simulink model "light_utility_aircraft_model"  (R2025b)
settings: Solver=ode23t  StartTime=0.0  StopTime=7800*4000  MaxStep=1000  RelTol=1e-1
values from neighbouring files (symbols this model uses):
  batt_temp = 273+25    (= 298)    [model_parameters.m]
  batt_volt = 647    [model_parameters.m]
  Cch = 3    [model_parameters.m]
  Cdch = 1    [model_parameters.m]
  Crate = 360    [model_parameters.m]
  max_SOC = 100    [model_parameters.m]
  min_SOC = 43    [model_parameters.m]
undefined symbols (not defined in the file or in its folder): battery_builder_rtmsupport, DiscIntNeverNeededParam, gcbh
libraries: nesl_utility x254, cylindricalPack_58kwhr_30Q_fade_lib x120, batt_lib x48, fl_lib x6, dspswit3 x2, simulink x1
(part 1 of 25)
settings Model: Creator=OADEBAJO  LastModifiedBy=oadebajo
settings DebuggingCC: AlgebraicLoopMsg=none

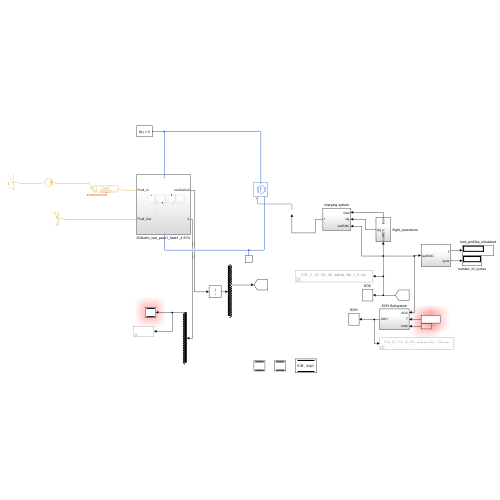
[thumbnail: Simulink's stored preview of the top-level diagram]
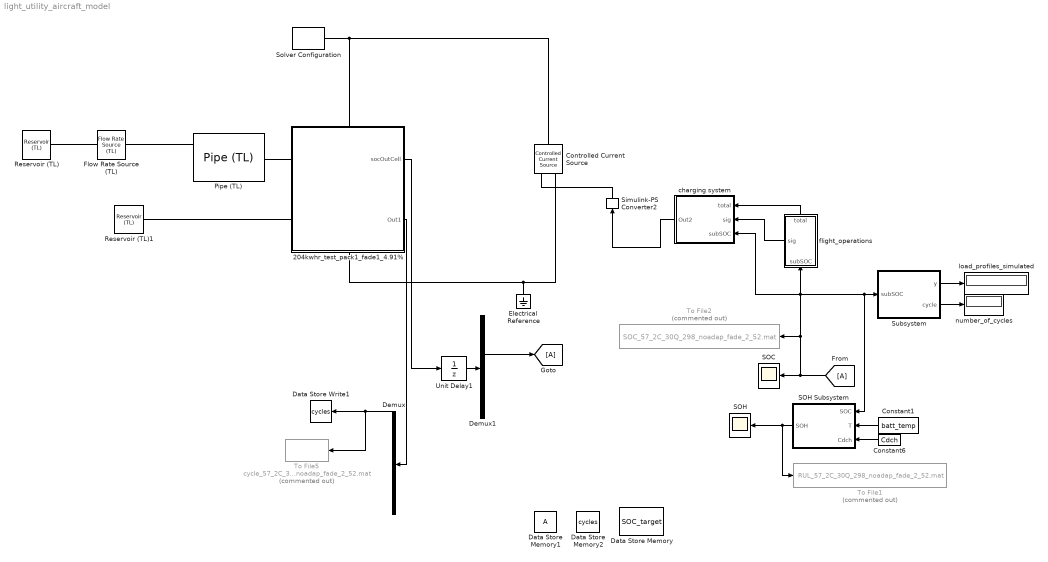
== system light_utility_aircraft_model ==
  204kwhr_test_pack1_fade1_4.91% : Subsystem
      settings: PortSchema="{\"entries\":[{\"content\":{\"sides\":[{\"content\":{\"connectorIds\":[\"LConn1\",\"LConn2\"]},\"type\":\"ConnectorPlacement.EquallySpacedRectSide\",\"uuid\":\"214b9fcb-0c97-4a4b-90ce-45ac7c4dd314\"},{\"content\":{\"connectorIds\":[\"Out1\",\"Out2\"],\"side\":\"RIGHT\"},\"type\":\"ConnectorPlacement.EquallySpacedRectSide\",\"uuid\":\"907ae7f8-29a0-40ea-b941-2e50330af598\"},{\"content\":{\"connectorIds\":[\"LConn3\"],\"side\":\"TOP\"},\"type\":\"ConnectorPlacement.EquallySpacedRectSide\",\"uuid\":\"96475e4e-0276-4503-89b2-fbbc8f8b3a57\"},{\"content\":{\"connectorIds\":[\"RConn1\"],\"side\":\"BOTTOM\"},\"type\":\"ConnectorPlacement.EquallySpacedRectSide\",\"uuid\":\"b4d79bed-ba89-429f-9016-8e0b58ea1225\"}]},\"type\":\"ConnectorPlacement.EquallySpacedConnector\",\"uuid\":\"7f0a58fe-ee0d-4bec-96b9-f1a67c994a61\"}],\"packageUris\":[\"http://schema.mathworks.com/mf0/Connector/R2020b\"],\"version\":\"1.0\"}"
  Constant1 : Constant  Value=batt_temp
  Constant6 : Constant  Value=Cdch
  Controlled Current Source : fl_lib/Electrical/Electrical Sources/Controlled Current Source
  Data Store Memory : DataStoreMemory  DataStoreName=SOC_target  WriteAfterWriteMsg=warning  ShowAdditionalParam=off
  Data Store Memory1 : DataStoreMemory  ReadBeforeWriteMsg=warning  WriteAfterWriteMsg=warning  WriteAfterReadMsg=warning  InitialValue=|  ShowAdditionalParam=off  OutDataTypeStr=double
      | [2000
      | 38016.66667
      | 37985.83333
      | 38114
      | 38470
      | 38050
      | 36925
      | 36686.66667
      | 36356.66667
      | 36665
      | 36675.83333
      | 36373.33333
      | 36550
      | 36696.66667
      | 36866.66667
      | 37022.5
      | 37191.33333
      | 37345
      | 37218
      | 37385.83333
      | 37170
      | 36890
      | 36601.33333
      | 36200.83333
      | 35166
      | 32305
      | 32462.66667
      | 31617.5
      | 31165.83333
      | 30523.33333
      | 30460
      | 30261.66667
      | 29913.33333
      | 30440
      | 31586.66667
      | 31543.33333
      | 31075
      | 30460
      | 30705
      | 31030
      | 31563.33333
      | 32118
      | 31650
      | 30480
      | 30505.83333
      | 31034
      | 31050
      | 31050
      | 31050
      | 31050
      | 30870
      | 30825
      | 30851.66667
      | 30460
      | 30300
      | 29880
      | 30050
      | 30460
      | 30460
      | 30508.33333
      | 30804
      | 30490
      | 30820
      | 30460
      | 30460
      | 30460
      | 30164
      | 29880
      | 30173.33333
      | 30460
      | 30460
      | 30293.33333
      | 30230
      | 30646.66667
      | 31427.5
      | 32174
      | 31580
      | 30460
      | 30512.5
      | 31060.66667
      | 31136.66667
      | 31200
      | 31283.33333
      | 31357.33333
      | 31430
      | 31504.66667
      | 31577.5
      | 31916
      | 32126.66667
      | 31566.66667
      | 31327.5
      | 31115.83333
      | 30533.33333
      | 30460
      | 30460
      | 30816.66667
      | 31050
      | 31050
      | 31050
      | 31000
      | 30270
      | 31118.33333
      | 31560
      | 31132.5
      | 31809.33333
      | 32210
      | 32210
      | 31656.66667
      | 31357.5
      | 30986.66667
      | 30753.33333
      | 31897.5
      | 31917.5
      | 30770
      | 31050
      | 30644
      | 29657.5
      | 30580
      | 30823.33333
      | 31196.66667
      | 29307.5
      | 29892.5
      | 26681.66667
      | 27240
      | 27540
      | 27362.66667
      | 26960
      | 26960
      | 26960
      | 26960
      | 26960
      | 26960
      | 27465
      | 27540
      | 27036.66667
      | 26960
      | 26960
      | 27295
      | 27540
      | 27540
      | 27396.66667
      | 26960
      | 27047.5
      | 27135
      | 26960
      | 27411.66667
      | 27540
      | 27030
      | 26570.66667
      | 28661.66667
      | 33762.5
      | 39203.33333
      | 60446.66667
      | 82117.5
      | 110829.1667
      | 110388
      | 116205
      | 118564
      | 118603.3333
      | 119512
      | 111716.6667
      | 83120
      | 66835
      | 34828.33333
      | 36093.33333
      | 33270
      | 31767.5
      | 31630
      | 31630
      | 31213.33333
      | 32210
      | 30717.5
      | 30460
      | 30460
      | 30460
      | 30460
      | 30816.66667
      | 30460
      | 30586
      | 31050
      | 30910
      | 30490
      | 30938.33333
      | 30460
      | 30528.33333
      | 30651.66667
      | 30560
      | 31025
      | 30479.33333
      | 30460
      | 30460
      | 30460
      | 30147.5
      | 30501.66667
      | 31023.33333
      | 30596
      | 30460
      | 30561.33333
      | 30755
      | 29981.33333
      | 29880
      | 29880
      | 29903.33333
      | 30418.33333
      | 30817.5
      | 30991.66667
      | 30460
      | 30460
      | 30460
      | 30460
      | 30950
      | 31050
      | 31050
      | 31050
      | 31050
      | 31050
      | 30600
      | 30460
      | 30818
      | 30460
      | 30816.66667
      | 31089.16667
      | 30940
      | 31676.66667
      | 31516.66667
      | 30427.5
      | 29880
      | 30251.66667
      | 30460
      | 30442
      | 29905.83333
      | 30350
      | 31010
      | 30538.33333
      | 30460
      | 31313.33333
      | 32093.33333
      | 33714
      | 34307.5
      | 33970
      | 33695
      | 33818.66667
      | 34481.66667
      | 34820
      | 59860
      | 76430
      | 151665
      | 154560.8333
      | 232958.6667
      | 231350
      | 232070
      | 231858.3333
      | 220890
      | 225815
      | 229102.5
      | 230615
      | 232486.6667
      | 233333.3333
      | 233500
      | 232154.1667
      | 230468
      | 228930
      | 229006.6667
      | 229836.6667
      | 230382.5
      | 223366.6667
      | 216663.3333
      | 204277.5
      | 189920.8333
      | 189770
      | 191450
      | 189118.6667
      | 189555.8333
      | 188840
      | 184448.3333
      | 183048.3333
      | 184440
      | 184956.6667
      | 184896.6667
      | 183412
      | 182658.3333
      | 179545.3333
      | 175645
      | 175086.6667
      | 170024.1667
      | 166452.5
      | 163130
      | 165680
      | 161725
      | 160212.5
      | 160422
      | 169190
      | 167867.3333
      | 168483.3333
      | 168900
      | 168465
      | 167872.6667
      | 166057.5
      | 166100
      | 168700
      | 167844
      | 168100
      | 167290
      | 167490
      | 165093.3333
      | 168485
      | 168115
      | 168623.3333
      | 169350
      | 171222
      | 171535
      | 171806.6667
      | 173960
      | 176183.3333
      | 176946.6667
      | 176810
      | 170711.6667
      | 172366.6667
      | 175735
      | 174870
      | 173703.3333
      | 173380
      | 174165.8333
      | 175250
      | 175250
      | 174833.3333
      | 174660
      | 174910
      | 174290
      | 173840
      | 173490
      | 173210.8333
      | 173260
      | 175740
      | 173692.6667
      | 172180.8333
      | 172418
      | 172330
      | 174247.3333
      | 174010
      | 174496.6667
      | 174240
      | 173790
      | 174280.8333
      | 173590
      | 174080
      | 174016.6667
      | 173242.5
      | 172537.5
      | 172866
      | 174051.6667
      | 174064
      | 173778.3333
      | 173918.6667
      | 174060
      | 173563.3333
      | 173038.3333
      | 172910
      | 173450
      | 173620
      | 173922.5
      | 172150
      | 174340
      | 174605
      | 174697.5
      | 174660
      | 174220
      | 174380
      | 174509.1667
      | 175035
      | 177580
      | 177213.3333
      | 173662
      | 174173.3333
      | 173629.3333
      | 173200
      | 173507.3333
      | 172515
      | 171608
      | 172198.3333
      | 173000.6667
      | 173232.5
      | 173450
      | 173680
      | 173885
      | 173599.1667
      | 173805
      | 175200
      | 173950
      | 173506.6667
      | 173697.5
      | 175117.3333
      | 175143.3333
      | 174102
      | 171750
      | 173811.3333
      | 174660
      | 174660.6667
      | 175020.8333
      | 173026
      | 173011.6667
      | 172330
      | 171440
      | 172716.6667
      | 172840
      | 172850
      | 173114.1667
      | 173911.6667
      | 174890
      | 174546.6667
      | 174070
      | 174195
      | 174638
      | 173948.3333
      | 174486
      | 174006.6667
      | 174064
      | 173510
      | 174560
      | 175118.3333
      | 175562
      | 174893.3333
      | 173742.6667
      | 173500
      | 174509.1667
      | 174076.6667
      | 174200
      | 174817.5
      | 174089.1667
      | 174580
      | 175066.6667
      | 174486.6667
      | 174987.5
      | 173500
      | 173693.3333
      | 172910
      | 172910
      | 173134
      | 174060
      | 172480.6667
      | 173487.5
      | 173838
      | 173936.6667
      | 172592.6667
      | 173405
      | 171750
      | 173043.3333
      | 173145
      | 172905.8333
      | 173580.8333
      | 174188
      | 173070
      | 172160
      | 173272.5
      | 174660
      | 173383.3333
      | 172790
      | 173135.8333
      | 172000.6667
      | 174390
      | 174966
      | 173070
      | 173750
      | 173060
      | 173200
      | 172330
      | 174398.3333
      | 175416.6667
      | 176185
      | 176485
      | 174518.3333
      | 174744
      | 172843.3333
      | 173766.6667
      | 173152.5
      | 175450
      | 174283.3333
      | 172910
      | 172915.8333
      | 172345.3333
      | 171750
      | 170495.3333
      | 169069.1667
      | 170326
      | 168965
      | 168830
      | 169212.5
      | 168517.5
      | 168590
      | 169265
      | 169484.6667
      | 169410
      | 169562
      | 168943.3333
      | 168823.3333
      | 168830
      | 168830
      | 168873.3333
      | 169480
      | 169907.5
      | 169426.6667
      | 168680
      | 168102
      | 169845.8333
      | 170252.5
      | 169485
      | 169980
      | 170772.5
      | 169399.1667
      | 168116.6667
      | 167990
      | 168240
      | 171195
      | 171728
      | 171350
      | 170880
      | 169835
      | 169605.8333
      | 167503.3333
      | 168120
      | 167605.8333
      | 168158
      | 169170
      | 168593.3333
      | 170163.3333
      | 173390
      | 173495
      | 173433.3333
      | 172575
      | 172915
      | 173290
      | 173295
      | 173544.6667
      | 172797.5
      | 172496
      | 171420
      | 172030
      | 171930
      | 171915.8333
      | 171133.3333
      | 169760
      | 171856.6667
      | 172175.3333
      | 174600
      | 172131.3333
      | 172020.8333
      | 172120
      | 171860
      | 173156.6667
      | 173735
      | 172939.1667
      | 173216
      | 172055
      | 172908
      | 173535
      | 173430
      | 173166.6667
      | 173220
      | 174190
      | 174660
      | 173180
      | 172650
      | 173004.1667
      | 172119.3333
      | 172910
      | 171673.3333
      | 171375
      | 171712.5
      | 172090
      | 171800
      | 172155
      | 172150
      | 171081.3333
      | 171120
      | 171700
      | 172665
      | 171172
      | 171866.6667
      | 172946.6667
      | 172520
      | 172784.1667
      | 171068.3333
      | 173300
      | 173575
      | 173638
      | 173160
      | 173581.6667
      | 174050.8333
      | 173915
      | 174215
      | 173573.3333
      | 174550
      | 173344.1667
      | 172577.3333
      | 172915
      | 171750
      | 172176.6667
      | 172642
      | 172153.3333
      | 173625
      | 172677.5
      | 172861.6667
      | 171396.6667
      | 171830
      | 172711.3333
      | 173547.5
      | 173300
      | 171933.3333
      | 172330
      | 172330
      | 172296.6667
      | 172510
      | 172276
      | 170840.8333
      | 172425.3333
      | 172650
      | 172637.3333
      | 173182.5
      | 172992.5
      | 173086.6667
      | 172876.6667
      | 173650
      | 173451.6667
      | 174288.3333
      | 174160
      | 174604.6667
      | 174315.8333
      | 171380
      | 172448.3333
      | 173700
      | 172890
      | 171913.3333
      | 172266.6667
      | 172204
      | 172184.1667
      | 172258
      | 172910
      | 173494.6667
      | 171160
      | 171597.5
      | 171380
      | 171338.3333
      | 170945
      | 171848.3333
      | 172528.6667
      | 171610
      | 170841.3333
      | 170914.1667
      | 172100
      | 172710
      | 170332.5
      | 171492.5
      | 172476.6667
      | 172910
      | 173252
      | 172918.3333
      | 172154
      | 172650
      | 171816.6667
      | 172024.1667
      | 171832.5
      | 171346.6667
      | 172776.6667
      | 172442.5
      | 172464.1667
      | 173161.3333
      | 173730
      | 173621.3333
      | 172357.5
      | 171420
      | 172786.6667
      | 172821.6667
      | 171702.5
      | 172596.6667
      | 170980
      | 172530
      | 172431.6667
      | 172250
      | 172330
      | 171826.6667
      | 172000
      | 153380
      | 146213.3333
      | 144631.6667
      | 141415
      | 141926.6667
      | 139505.3333
      | 135980
      | 129564.6667
      | 125999.1667
      | 123940
      | 123438.3333
      | 122295.3333
      | 116815
      | 117846.6667
      | 115203.3333
      | 115708
      | 116565.8333
      | 115455.3333
      | 113510
      | 112726.6667
      | 111845.8333
      | 108307.5
      | 96923.33333
      | 86050
      | 75792
      | 73804.16667
      | 74118.66667
      | 73000
      | 72070
      | 70140.83333
      | 67820
      | 60416.66667
      | 59270
      | 60102.5
      | 59760
      | 59180
      | 58600
      | 61015.83333
      | 64317.5
      | 69340
      | 71306.66667
      | 64695.83333
      | 60487.5
      | 40273.33333
      | 34888.33333
      | 31640
      | 27380
      | 25226
      | 23600
      | 24616
      | 25837.5
      | 26462
      | 26331.66667
      | 26380
      | 26905
      | 26543.33333
      | 26703.33333
      | 26016
      | 27002.5
      | 27540
      | 26730
      | 26573.33333
      | 26652.5
      | 26980
      | 27540
      | 27115
      | 27840
      | 28698.33333
      | 32944
      | 31090
      | 32941.33333
      | 33380
      | 33380
      | 31378.33333
      | 32210
      | 31247.5
      | 28076.66667
      | 28710
      | 28710
      | 27811.66667
      | 28130
      | 28130
      | 27800
      | 28675
      | 28565
      | 28444
      | 28330
      | 28208
      | 28095
      | 27962
      | 27850
      | 27738
      | 27615
      | 27870
      | 28130
      | 28130
      | 28130
      | 27730
      | 27540
      | 27540
      | 27540
      | 27540
      | 27755
      | 28070
      | 27540
      | 27540
      | 27540
      | 27540
      | 27540
      | 27540
      | 16000
      | 2000]
  Data Store Memory2 : DataStoreMemory  DataStoreName=cycles  WriteAfterWriteMsg=warning  ShowAdditionalParam=off
  Data Store Write1 : DataStoreWrite  DataStoreName=cycles  IndexOptions=Index vector (dialog)  Indices=1  OutputSizes=1
  Demux : Demux  Outputs=30
  Demux1 : Demux  Outputs=30
  Electrical Reference : fl_lib/Electrical/Electrical Elements/Electrical Reference
  Flow Rate Source (TL) : fl_lib/Thermal Liquid/Sources/Flow Rate Source (TL)  source_type=foundation.enum.constant_controlled.constant  flow_type=foundation.enum.mass_volumetric_flow.mass  mass_flow=1.75 kg/s  volumetric_flow=0 m^3/s  power_spec=foundation.enum.power_spec.isentropic  area=0.01 m^2
      settings: InternalSimscapePortConfiguration="{\"Bottom\":[],\"Left\":[{\"id\":\"A\",\"label\":\"A\",\"type\":\"foundation.thermal_liquid.thermal_liquid\"}],\"Right\":[{\"id\":\"B\",\"label\":\"B\",\"type\":\"foundation.thermal_liquid.thermal_liquid\"}],\"Top\":[]}"
  From : From
  Goto : Goto
  Pipe (TL) : fl_lib/Thermal Liquid/Elements/Pipe (TL)  length=5 m  area=0.01 m^2  Dh=0.1128 m  length_add=1 m  roughness=15e-6 m  Re_lam=2000  Re_tur=4000  shape_factor=64  Nu_lam=3.66  dynamic_compressibility=true  inertia=false  p0=0.101325 MPa  T0=293.15 K  mdot0=0 kg/s  p_nominal=0.101325 MPa  T_nominal=293.15 K
  Reservoir (TL) : fl_lib/Thermal Liquid/Elements/Reservoir (TL)  pressure_spec=foundation.enum.pressure_spec.atmospheric  reservoir_pressure=0.101325 MPa  reservoir_temperature=298 K  area=0.01 m^2
  Reservoir (TL)1 : fl_lib/Thermal Liquid/Elements/Reservoir (TL)  pressure_spec=foundation.enum.pressure_spec.atmospheric  reservoir_pressure=0.101325 MPa  reservoir_temperature=298 K  area=0.01 m^2
  SOC : Scope
      settings: ActiveDisplayYMinimum=-0.12565653108728628  ActiveDisplayYMaximum=1.1309087797855768  DataLoggingVariableName=ScopeData4
  SOH : Scope
      settings: ActiveDisplayYMinimum=63.077667459608676  ActiveDisplayYMaximum=104.10248139337682  DataLoggingVariableName=ScopeData3
  SOH Subsystem : Subsystem
  Simulink-PS Converter2 : nesl_utility/Simulink-PS Converter  PhysicalDomain=network_engine_domain  SubClassName=ps_input  LeftPortType=input  RightPortType=output  PseudoPeriodic=off  Frequencies=[]  NoiseDistribution=none  NoiseParameters=[]  Unit=1  AffineConversion=off  FilteringAndDerivatives=provide  SimscapeFilterOrder=1  InputFilterTimeConstant=0.001  UdotUserProvided=0
  Solver Configuration : nesl_utility/Solver Configuration  PhysicalDomain=network_engine_domain  SubClassName=solver  LeftPortType=input  RightPortType=generic  Tones=[]  Harmonics=[]  SimulateNoise=off  Profile=off  UseLocalSampling=off  EnableSwitchedLinearOptims=on  FrequencyDomain=off  UseCCode=off  RelTol=0.001  AbsTol=0.001  MinStep=1e-09  ToWorkspace=off  RevertToSquareIcSolve=off  NumHomotopyIterations=100  PhasorMode=off  PhasorModeNumHarmonics=1  ConsistencySolver=NEWTON_XTOL_AFTER_FTOL  IndexReductionMethod=DerivativeReplacement  DoDC=off  ResidualTolerance=1e-09  ConsistencyTolSource=GLOBAL  ConsistencyAbsTol=0.1  ConsistencyRelTol=0.1  ConsistencyTolFactor=0.1  UseLocalSolver=on  LocalSolverChoice=NE_BACKWARD_EULER_ADVANCER  ResolveIndetEquations=on  FunctionEvalNumThread=1  LocalSolverSampleTime=20  DoFixedCost=off  MaxNonlinIter=3  MaxModeIter=2  ComputeImpulses=off  ImpulseIterations=2  LinearAlgebra=auto  LinearAlgebraNumThread=0  EquationFormulation=NE_TIME_EF  DelaysMemoryBudget=1024  AutomaticFiltering=off  FilteringTimeConstant=0.001  PartitionStorageMethod=AS_NEEDED  PartitionMemoryBudget=1024  PartitionMethod=ROBUST  MultibodyUseLocalSolver=off  MultibodyLocalSolverChoice=ODE1  MultibodyLocalSolverSampleTime=0.001
  Subsystem : Subsystem
  To File1 : ToFile  Commented=on  Filename=RUL_57_2C_30Q_298_noadap_fade_2_52.mat  SaveFormat=Timeseries
  To File2 : ToFile  Commented=on  Filename=SOC_57_2C_30Q_298_noadap_fade_2_52.mat  SaveFormat=Timeseries
  To File5 : ToFile  Commented=on  Filename=cycle_57_2C_30Q_298_noadap_fade_2_52.mat  SaveFormat=Timeseries
  Unit Delay1 : UnitDelay  SampleTime=-1  HasFrameUpgradeWarning=on
  charging system : Subsystem
  flight_operations : Subsystem
      settings: PortSchema="{\"entries\":[{\"content\":{\"sides\":[{\"content\":{\"connectorIds\":[\"In1\"]},\"type\":\"ConnectorPlacement.EquallySpacedRectSide\",\"uuid\":\"b096ff91-cb2d-4b3b-84bd-326afe2dcca0\"},{\"content\":{\"connectorIds\":[\"Out1\"],\"side\":\"RIGHT\"},\"type\":\"ConnectorPlacement.EquallySpacedRectSide\",\"uuid\":\"96e6b261-4fad-4682-8891-262764578e26\"},{\"content\":{\"connectorIds\":[\"Out2\"],\"side\":\"TOP\"},\"type\":\"ConnectorPlacement.EquallySpacedRectSide\",\"uuid\":\"cf441717-ac42-41e6-9847-085a0e0f05ac\"}]},\"type\":\"ConnectorPlacement.EquallySpacedConnector\",\"uuid\":\"ee456057-6485-49ed-8376-ab99d7c41737\"}],\"packageUris\":[\"http://schema.mathworks.com/mf0/Connector/R2020b\"],\"version\":\"1.0\"}"
  load_profiles_simulated : Display
  number_of_cycles : Display
  wires:
  SOH Subsystem/1(SOH) -> To File1/1, SOH/1
  Constant6/1 -> SOH Subsystem/3(Cdch)
  Subsystem/1(y) -> load_profiles_simulated/1
  Subsystem/2(cycle) -> number_of_cycles/1
  204kwhr_test_pack1_fade1_4.91%/1(socOutCell) -> Unit Delay1/1
  Demux1/12 -> Goto/1
  Constant1/1 -> SOH Subsystem/2(T)
  Demux/1 -> To File5/1, Data Store Write1/1
  From/1 -> charging system/3(subSOC), flight_operations/1(subSOC), SOH Subsystem/1(SOC), Subsystem/1(subSOC), To File2/1, SOC/1
  204kwhr_test_pack1_fade1_4.91%/2(Out1) -> Demux/1
  Unit Delay1/1 -> Demux1/1
  charging system/1(Out2) -> Simulink-PS Converter2/1
  flight_operations/2(sig) -> charging system/2(sig)
  flight_operations/1(total) -> charging system/1(total)
  connections:
  Simulink-PS Converter2/RConn1 -- Controlled Current Source/RConn1
  Flow Rate Source (TL)/LConn1 -- Reservoir (TL)/LConn1
  Pipe (TL)/LConn1 -- Flow Rate Source (TL)/RConn1
  Solver Configuration/RConn1 -- Controlled Current Source/LConn1 -- 204kwhr_test_pack1_fade1_4.91%/LConn3
  Controlled Current Source/RConn2 -- 204kwhr_test_pack1_fade1_4.91%/RConn1 -- Electrical Reference/LConn1
  Pipe (TL)/RConn1 -- 204kwhr_test_pack1_fade1_4.91%/LConn1
  Reservoir (TL)1/LConn1 -- 204kwhr_test_pack1_fade1_4.91%/LConn2

== system light_utility_aircraft_model/204kwhr_test_pack1_fade1_4.91% ==
  Pack1 : Subsystem  LoadFcn=battery_builder_rtmsupport('loadfcn',gcbh);
      settings: CopyFcn=battery_builder_rtmsupport('copyfcn',gcbh);  PreCopyFcn=battery_builder_rtmsupport('precopyfcn',gcbh);  PreDeleteFcn=battery_builder_rtmsupport('predeletefcn',gcbh);  PortSchema="{\"entries\":[{\"content\":{\"sides\":[{\"content\":{\"connectorIds\":[\"LConn1\"]},\"type\":\"ConnectorPlacement.EquallySpacedRectSide\",\"uuid\":\"4c3e3939-f290-4977-b563-9b18c075fb3a\"},{\"content\":{\"connectorIds\":[\"Out1\",\"RConn1\"],\"side\":\"RIGHT\"},\"type\":\"ConnectorPlacement.EquallySpacedRectSide\",\"uuid\":\"15891230-3eac-4f5c-b3ce-979a3f3926a0\"},{\"content\":{\"connectorIds\":[\"LConn2\",\"In1\",\"LConn3\"],\"side\":\"TOP\"},\"type\":\"ConnectorPlacement.EquallySpacedRectSide\",\"uuid\":\"7ff468eb-bac1-4195-909a-c5f40a7166b0\"},{\"content\":{\"connectorIds\":[\"Out2\",\"Out3\",\"Out4\",\"Out5\",\"Out6\",\"Out7\",\"Out8\",\"Out9\"],\"side\":\"BOTTOM\"},\"type\":\"ConnectorPlacement.EquallySpacedRectSide\",\"uuid\":\"9665d171-8307-4bd8-a9b3-8f2ee197f547\"}]},\"type\":\"ConnectorPlacement.EquallySpacedConnector\",\"uuid\":\"e275b05f-d8a8-44f7-ab25-c2ce0bf8487f\"}],\"packageUris\":[\"http://schema.mathworks.com/mf0/Connector/R2020b\"],\"version\":\"1.0\"}"
      mask Display: image('$imagefile')
  Pack2 : Subsystem  LoadFcn=battery_builder_rtmsupport('loadfcn',gcbh);
      settings: CopyFcn=battery_builder_rtmsupport('copyfcn',gcbh);  PreCopyFcn=battery_builder_rtmsupport('precopyfcn',gcbh);  PreDeleteFcn=battery_builder_rtmsupport('predeletefcn',gcbh);  PortSchema="{\"entries\":[{\"content\":{\"sides\":[{\"content\":{\"connectorIds\":[\"LConn1\"]},\"type\":\"ConnectorPlacement.EquallySpacedRectSide\",\"uuid\":\"4c3e3939-f290-4977-b563-9b18c075fb3a\"},{\"content\":{\"connectorIds\":[\"Out1\",\"RConn1\"],\"side\":\"RIGHT\"},\"type\":\"ConnectorPlacement.EquallySpacedRectSide\",\"uuid\":\"15891230-3eac-4f5c-b3ce-979a3f3926a0\"},{\"content\":{\"connectorIds\":[\"LConn2\",\"In1\",\"LConn3\"],\"side\":\"TOP\"},\"type\":\"ConnectorPlacement.EquallySpacedRectSide\",\"uuid\":\"7ff468eb-bac1-4195-909a-c5f40a7166b0\"},{\"content\":{\"connectorIds\":[\"Out2\",\"Out3\",\"Out4\",\"Out5\",\"Out6\",\"Out7\",\"Out8\",\"Out9\"],\"side\":\"BOTTOM\"},\"type\":\"ConnectorPlacement.EquallySpacedRectSide\",\"uuid\":\"9665d171-8307-4bd8-a9b3-8f2ee197f547\"}]},\"type\":\"ConnectorPlacement.EquallySpacedConnector\",\"uuid\":\"e275b05f-d8a8-44f7-ab25-c2ce0bf8487f\"}],\"packageUris\":[\"http://schema.mathworks.com/mf0/Connector/R2020b\"],\"version\":\"1.0\"}"
      mask Display: image('pack_1.JPG');
  Pack3 : Subsystem  LoadFcn=battery_builder_rtmsupport('loadfcn',gcbh);
      settings: CopyFcn=battery_builder_rtmsupport('copyfcn',gcbh);  PreCopyFcn=battery_builder_rtmsupport('precopyfcn',gcbh);  PreDeleteFcn=battery_builder_rtmsupport('predeletefcn',gcbh);  PortSchema="{\"entries\":[{\"content\":{\"sides\":[{\"content\":{\"connectorIds\":[\"LConn1\"]},\"type\":\"ConnectorPlacement.EquallySpacedRectSide\",\"uuid\":\"4c3e3939-f290-4977-b563-9b18c075fb3a\"},{\"content\":{\"connectorIds\":[\"Out1\",\"RConn1\"],\"side\":\"RIGHT\"},\"type\":\"ConnectorPlacement.EquallySpacedRectSide\",\"uuid\":\"15891230-3eac-4f5c-b3ce-979a3f3926a0\"},{\"content\":{\"connectorIds\":[\"LConn2\",\"In1\",\"LConn3\"],\"side\":\"TOP\"},\"type\":\"ConnectorPlacement.EquallySpacedRectSide\",\"uuid\":\"7ff468eb-bac1-4195-909a-c5f40a7166b0\"},{\"content\":{\"connectorIds\":[\"Out2\",\"Out3\",\"Out4\",\"Out5\",\"Out6\",\"Out7\",\"Out8\",\"Out9\"],\"side\":\"BOTTOM\"},\"type\":\"ConnectorPlacement.EquallySpacedRectSide\",\"uuid\":\"9665d171-8307-4bd8-a9b3-8f2ee197f547\"}]},\"type\":\"ConnectorPlacement.EquallySpacedConnector\",\"uuid\":\"e275b05f-d8a8-44f7-ab25-c2ce0bf8487f\"}],\"packageUris\":[\"http://schema.mathworks.com/mf0/Connector/R2020b\"],\"version\":\"1.0\"}"
      mask Display: image('pack_2.JPG');
  Pack4 : Subsystem  LoadFcn=battery_builder_rtmsupport('loadfcn',gcbh);
      settings: CopyFcn=battery_builder_rtmsupport('copyfcn',gcbh);  PreCopyFcn=battery_builder_rtmsupport('precopyfcn',gcbh);  PreDeleteFcn=battery_builder_rtmsupport('predeletefcn',gcbh);  PortSchema="{\"entries\":[{\"content\":{\"sides\":[{\"content\":{\"connectorIds\":[\"LConn1\"]},\"type\":\"ConnectorPlacement.EquallySpacedRectSide\",\"uuid\":\"4c3e3939-f290-4977-b563-9b18c075fb3a\"},{\"content\":{\"connectorIds\":[\"Out1\",\"RConn1\"],\"side\":\"RIGHT\"},\"type\":\"ConnectorPlacement.EquallySpacedRectSide\",\"uuid\":\"15891230-3eac-4f5c-b3ce-979a3f3926a0\"},{\"content\":{\"connectorIds\":[\"LConn2\",\"In1\",\"LConn3\"],\"side\":\"TOP\"},\"type\":\"ConnectorPlacement.EquallySpacedRectSide\",\"uuid\":\"7ff468eb-bac1-4195-909a-c5f40a7166b0\"},{\"content\":{\"connectorIds\":[\"Out2\",\"Out3\",\"Out4\",\"Out5\",\"Out6\",\"Out7\",\"Out8\",\"Out9\"],\"side\":\"BOTTOM\"},\"type\":\"ConnectorPlacement.EquallySpacedRectSide\",\"uuid\":\"9665d171-8307-4bd8-a9b3-8f2ee197f547\"}]},\"type\":\"ConnectorPlacement.EquallySpacedConnector\",\"uuid\":\"e275b05f-d8a8-44f7-ab25-c2ce0bf8487f\"}],\"packageUris\":[\"http://schema.mathworks.com/mf0/Connector/R2020b\"],\"version\":\"1.0\"}"
      mask Display: image('pack_3.JPG');
  Terminator{,1..29} (30 alike) : Terminator
  Fluid_In : PMIOPort  Side=Left
  Fluid_Out : PMIOPort  Port=2  Side=Left
  + : PMIOPort  Port=3  Side=Left
  - : PMIOPort  Port=4  Side=Right
  socOutCell : Outport
  Out1 : Outport  Port=2
  wires:
  Pack1/4(numCyclesCell) -> Out1/1
  Pack1/2(socOutCell) -> socOutCell/1
  Pack1/3(iCell) -> Terminator/1
  Pack1/9(vParallelAssembly) -> Terminator5/1
  Pack1/8(vCell) -> Terminator4/1
  Pack1/7(temperatureCell) -> Terminator3/1
  Pack1/6(socParallelAssembly) -> Terminator2/1
  Pack1/5(socCell) -> Terminator1/1
  Pack2/9(vParallelAssembly) -> Terminator11/1
  Pack2/8(vCell) -> Terminator10/1
  Pack2/7(temperatureCell) -> Terminator9/1
  Pack2/6(socParallelAssembly) -> Terminator8/1
  Pack2/5(socCell) -> Terminator7/1
  Pack2/4(numCyclesCell) -> Terminator6/1
  Pack2/2(socOutCell) -> Terminator13/1
  Pack2/3(iCell) -> Terminator12/1
  Pack3/9(vParallelAssembly) -> Terminator15/1
  Pack3/8(vCell) -> Terminator14/1
  Pack3/7(temperatureCell) -> Terminator21/1
  Pack3/6(socParallelAssembly) -> Terminator20/1
  Pack3/5(socCell) -> Terminator19/1
  Pack3/4(numCyclesCell) -> Terminator18/1
  Pack3/3(iCell) -> Terminator16/1
  Pack3/2(socOutCell) -> Terminator17/1
  Pack4/2(socOutCell) -> Terminator25/1
  Pack4/3(iCell) -> Terminator24/1
  Pack4/4(numCyclesCell) -> Terminator26/1
  Pack4/5(socCell) -> Terminator27/1
  Pack4/6(socParallelAssembly) -> Terminator28/1
  Pack4/7(temperatureCell) -> Terminator29/1
  Pack4/8(vCell) -> Terminator22/1
  Pack4/9(vParallelAssembly) -> Terminator23/1
  connections:
  Pack2/RConn1 -- Pack1/RConn1 -- -/RConn1 -- Pack3/RConn1 -- Pack4/RConn1
  Pack2/LConn3 -- Pack1/LConn3 -- Fluid_Out/RConn1 -- Pack3/LConn3 -- Pack4/LConn3
  Pack2/LConn2 -- Pack1/LConn2 -- Fluid_In/RConn1 -- Pack3/LConn2 -- Pack4/LConn2
  +/RConn1 -- Pack1/LConn1 -- Pack2/LConn1 -- Pack3/LConn1 -- Pack4/LConn1

== system light_utility_aircraft_model/SOH Subsystem ==
  SOC : Inport
  T : Inport  Port=2
  Cdch : Inport  Port=3
  Constant1 : Constant  Value=Cch
  Constant2 : Constant  Value=min_SOC
  Constant3 : Constant  Value=max_SOC
  Data Store Read : DataStoreRead  DataStoreName=cycles  IndexOptions=Index vector (dialog)  Indices=1  OutputSizes=1
  Subsystem : Subsystem  Commented=on
  degradation constant : MATLAB Function  TreatAsAtomicUnit=on
  SOH : Outport
  wires:
  SOC/1 -> Subsystem/1(SOC)
  Data Store Read/1 -> degradation constant/1(FEC)
  Constant3/1 -> degradation constant/5(maxSOC)
  degradation constant/1(SOH) -> SOH/1  "SOH"
  Cdch/1 -> degradation constant/2(Cdch)
  Constant1/1 -> degradation constant/6(Cch)
  Constant2/1 -> degradation constant/4(minSOC)
  T/1 -> degradation constant/3(T)

== system light_utility_aircraft_model/Subsystem ==
  subSOC : Inport
  Scope29 : Scope
      settings: ActiveDisplayYMinimum=-1  ActiveDisplayYMaximum=1  DataLoggingVariableName=ScopeData7
  Subsystem4 : Subsystem
  cycle counter : Subsystem
  y : Outport
  cycle : Outport  Port=2
  wires:
  subSOC/1 -> cycle counter/1(subSOC)
  cycle counter/1(cnt) -> y/1, Subsystem4/1(y)
  Subsystem4/1(cycle) -> cycle/1, Scope29/1

== system light_utility_aircraft_model/charging system ==
  total : Inport
  sig : Inport  Port=2
  subSOC : Inport  Port=3
  Add2 : Sum
  Scope : Scope  NumInputPorts=2
  charge regulation1 : Subsystem
  power_to_current : Gain  Gain=-1/batt_volt
  Out2 : Outport
  wires:
  power_to_current/1 -> Scope/1, Add2/2
  Add2/1 -> Out2/1
  total/1 -> Scope/2, power_to_current/1
  charge regulation1/1(y) -> Add2/1
  sig/1 -> charge regulation1/2(sig)
  subSOC/1 -> charge regulation1/1(subSOC)

== system light_utility_aircraft_model/flight_operations ==
  subSOC : Inport
  Sum1 : Sum  Inputs=|++
  base_adjustment : MATLAB Function  TreatAsAtomicUnit=on
  profile_iteration : Subsystem
  total : Outport
  sig : Outport  Port=2
  wires:
  Sum1/1 -> total/1  "total"
  profile_iteration/1(sig) -> Sum1/1, base_adjustment/1(sig), sig/1
  profile_iteration/2(Out2) -> base_adjustment/2(t)
  base_adjustment/1(y) -> Sum1/2  "balancer"
  subSOC/1 -> profile_iteration/1(subSOC)
